simulink subsystem "ButtonStatesRF"  (R2023b) (continued)
== stateflow chart ButtonStatesRF/ButtonStates/buttonFlowChart == (continued)
                  Flow.ButtonTriggeredMenu.Menu.MainSubMenu.SettingsSubMenu.FavoritesSubMenu.Slot3Menu.Slot3FavoritesMenu.Slot3Connectivity -> Flow.ButtonTriggeredMenu.Menu.MainSubMenu.SettingsSubMenu.FavoritesSubMenu.Slot3Menu.Slot3FavoritesMenu.Slot3Odometer [order 1] : UP
                  (junction 6615) -> Flow.ButtonTriggeredMenu.Menu.MainSubMenu.SettingsSubMenu.FavoritesSubMenu.Slot3Menu.Slot3FavoritesMenu.Slot3Connectivity
                  Flow.ButtonTriggeredMenu.Menu.MainSubMenu.SettingsSubMenu.FavoritesSubMenu.Slot3Menu.Slot3FavoritesMenu.Slot3Connectivity -> (junction 6614) [order 2] : DOWN
                state favSlot3Updater(currState) [function]
                  (default) -> (junction 6665) : %{tempSlotSel  = eStateName.FAVORITES_TRIP_DISTANCE_1;}
                  (junction 6665) -> (junction 6666) [order 1] : [currState == favSlot1Sel ]
                  (junction 6666) -> (junction 6667) : { favSlot1Sel = favSlot3Sel;
                      | BLU_FnSaveSlot1Fav(favSlot1Sel);}
                  (junction 6665) -> (junction 6668) [order 2]
                  (junction 6667) -> (junction 6670)
                  (junction 6668) -> (junction 6669) [order 1] : [currState == favSlot2Sel]
                  (junction 6669) -> (junction 6670) : {favSlot2Sel = favSlot3Sel;
                      | BLU_FnSaveSlot2Fav(favSlot2Sel);}
                  (junction 6668) -> (junction 6671) [order 2]
                  (junction 6670) -> (junction 6673)
                  (junction 6671) -> (junction 6672) [order 1] : [currState == favSlot4Sel ]
                  (junction 6672) -> (junction 6673) : {favSlot4Sel = favSlot3Sel;
                      | BLU_FnSaveSlot4Fav(favSlot4Sel);}
                  (junction 6671) -> (junction 6674) [order 2]
                  (junction 6673) -> (junction 6675)
                  (junction 6675) -> (junction 6674)
                  (junction 6674) -> (junction 6676) : {favSlot3Sel = currState;
                      | BLU_FnSaveSlot3Fav(favSlot3Sel);}
                state Slot3FavoritesMenu1 [commented out]
                  state Slot3TripDistance [commented out]
                    en:
                    currentState = eStateName.FAVORITES_TRIP_DISTANCE_1;
                  state Slot3AverageSpeed [commented out]
                    en:
                    currentState = eStateName.FAVORITES_AVERAGE_SPEED_1;
                  state Slot3AverageFuelCons [commented out]
                    en:
                    currentState = eStateName.FAVORITES_AVERAGE_FUEL_CONS_1;
                  state Slot3TripTime [commented out]
                    en:
                    currentState = eStateName.FAVORITES_TRIP_TIME_1;
                  state Slot3AverageTripDistance [commented out]
                    en:
                    currentState = eStateName.FAVORITES_TRIP_DISTANCE_2;
                  state Slot3AverageSpeed1 [commented out]
                    en:
                    currentState = eStateName.FAVORITES_AVERAGE_SPEED_2;
                  state Slot3AverageFuelCons1 [commented out]
                    en:
                    currentState = eStateName.FAVORITES_AVERAGE_FUEL_CONS_2;
                  state Slot3TripTime2 [commented out]
                    en:
                    currentState = eStateName.FAVORITES_TRIP_TIME_2;
                  state Slot3FuelRange [commented out]
                    en:
                    currentState = eStateName.FAVORITES_FUEL_RANGE;
                  state Slot3Date [commented out]
                    en:
                    currentState = eStateName.FAVORITES_DATE;
                  state Slot3Battery [commented out]
                    en:
                    currentState = eStateName.FAVORITES_BATTERY;
                  state Slot3WaterTemperature [commented out]
                    en:
                    currentState = eStateName.FAVORITES_WATER_TEMPERATURE;
                  state Slot3Odometer [commented out]
                    en:
                    currentState = eStateName.FAVORITES_ODOMETER;
                  state Slot3Connectivity [commented out]
                    en:
                    currentState = eStateName.FAVORITES_CONNECTIVITY;
                  (default) -> (junction 8899) [commented out]
                  (junction 8899) -> (junction 8897) [commented out] : [favSlot3Sel ==eStateName.FAVORITES_TRIP_DISTANCE_1 ]
                  (junction 8897) -> Flow.ButtonTriggeredMenu.Menu.MainSubMenu.SettingsSubMenu.FavoritesSubMenu.Slot3Menu.Slot3FavoritesMenu1.Slot3TripDistance [commented out]
                  Flow.ButtonTriggeredMenu.Menu.MainSubMenu.SettingsSubMenu.FavoritesSubMenu.Slot3Menu.Slot3FavoritesMenu1.Slot3TripDistance -> (junction 8898) [commented out] : UP
                  (junction 8898) -> (junction 8913) [commented out]
                  (junction 8899) -> (junction 8900) [commented out]
                  (junction 8914) -> (junction 8897) [commented out]
                  Flow.ButtonTriggeredMenu.Menu.MainSubMenu.SettingsSubMenu.FavoritesSubMenu.Slot3Menu.Slot3FavoritesMenu1.Slot3TripDistance -> Flow.ButtonTriggeredMenu.Menu.MainSubMenu.SettingsSubMenu.FavoritesSubMenu.Slot3Menu.Slot3FavoritesMenu1.Slot3AverageSpeed [commented out] : DOWN
                  Flow.ButtonTriggeredMenu.Menu.MainSubMenu.SettingsSubMenu.FavoritesSubMenu.Slot3Menu.Slot3FavoritesMenu1.Slot3AverageSpeed -> Flow.ButtonTriggeredMenu.Menu.MainSubMenu.SettingsSubMenu.FavoritesSubMenu.Slot3Menu.Slot3FavoritesMenu1.Slot3TripDistance [commented out] : UP
                  (junction 8900) -> Flow.ButtonTriggeredMenu.Menu.MainSubMenu.SettingsSubMenu.FavoritesSubMenu.Slot3Menu.Slot3FavoritesMenu1.Slot3AverageSpeed [commented out] : [favSlot3Sel ==eStateName.FAVORITES_AVERAGE_SPEED_1 ]
                  (junction 8900) -> (junction 8901) [commented out]
                  Flow.ButtonTriggeredMenu.Menu.MainSubMenu.SettingsSubMenu.FavoritesSubMenu.Slot3Menu.Slot3FavoritesMenu1.Slot3AverageSpeed -> Flow.ButtonTriggeredMenu.Menu.MainSubMenu.SettingsSubMenu.FavoritesSubMenu.Slot3Menu.Slot3FavoritesMenu1.Slot3AverageFuelCons [commented out] : DOWN
                  Flow.ButtonTriggeredMenu.Menu.MainSubMenu.SettingsSubMenu.FavoritesSubMenu.Slot3Menu.Slot3FavoritesMenu1.Slot3AverageFuelCons -> Flow.ButtonTriggeredMenu.Menu.MainSubMenu.SettingsSubMenu.FavoritesSubMenu.Slot3Menu.Slot3FavoritesMenu1.Slot3AverageSpeed [commented out] : UP
                  (junction 8901) -> Flow.ButtonTriggeredMenu.Menu.MainSubMenu.SettingsSubMenu.FavoritesSubMenu.Slot3Menu.Slot3FavoritesMenu1.Slot3AverageFuelCons [commented out] : [favSlot3Sel ==eStateName.FAVORITES_AVERAGE_FUEL_CONS_1 ]
                  (junction 8901) -> (junction 8902) [commented out]
                  Flow.ButtonTriggeredMenu.Menu.MainSubMenu.SettingsSubMenu.FavoritesSubMenu.Slot3Menu.Slot3FavoritesMenu1.Slot3AverageFuelCons -> Flow.ButtonTriggeredMenu.Menu.MainSubMenu.SettingsSubMenu.FavoritesSubMenu.Slot3Menu.Slot3FavoritesMenu1.Slot3TripTime [commented out] : DOWN
                  Flow.ButtonTriggeredMenu.Menu.MainSubMenu.SettingsSubMenu.FavoritesSubMenu.Slot3Menu.Slot3FavoritesMenu1.Slot3TripTime -> Flow.ButtonTriggeredMenu.Menu.MainSubMenu.SettingsSubMenu.FavoritesSubMenu.Slot3Menu.Slot3FavoritesMenu1.Slot3AverageFuelCons [commented out] : UP
                  (junction 8902) -> Flow.ButtonTriggeredMenu.Menu.MainSubMenu.SettingsSubMenu.FavoritesSubMenu.Slot3Menu.Slot3FavoritesMenu1.Slot3TripTime [commented out] : [favSlot3Sel ==eStateName.FAVORITES_TRIP_TIME_1 ]
                  (junction 8902) -> (junction 8903) [commented out]
                  Flow.ButtonTriggeredMenu.Menu.MainSubMenu.SettingsSubMenu.FavoritesSubMenu.Slot3Menu.Slot3FavoritesMenu1.Slot3TripTime -> Flow.ButtonTriggeredMenu.Menu.MainSubMenu.SettingsSubMenu.FavoritesSubMenu.Slot3Menu.Slot3FavoritesMenu1.Slot3AverageTripDistance [commented out] : DOWN
                  Flow.ButtonTriggeredMenu.Menu.MainSubMenu.SettingsSubMenu.FavoritesSubMenu.Slot3Menu.Slot3FavoritesMenu1.Slot3AverageTripDistance -> Flow.ButtonTriggeredMenu.Menu.MainSubMenu.SettingsSubMenu.FavoritesSubMenu.Slot3Menu.Slot3FavoritesMenu1.Slot3TripTime [commented out] : UP
                  (junction 8903) -> Flow.ButtonTriggeredMenu.Menu.MainSubMenu.SettingsSubMenu.FavoritesSubMenu.Slot3Menu.Slot3FavoritesMenu1.Slot3AverageTripDistance [commented out] : [favSlot3Sel ==eStateName.FAVORITES_TRIP_DISTANCE_2 ]
                  (junction 8903) -> (junction 8904) [commented out]
                  Flow.ButtonTriggeredMenu.Menu.MainSubMenu.SettingsSubMenu.FavoritesSubMenu.Slot3Menu.Slot3FavoritesMenu1.Slot3AverageTripDistance -> Flow.ButtonTriggeredMenu.Menu.MainSubMenu.SettingsSubMenu.FavoritesSubMenu.Slot3Menu.Slot3FavoritesMenu1.Slot3AverageSpeed1 [commented out] : DOWN
                  Flow.ButtonTriggeredMenu.Menu.MainSubMenu.SettingsSubMenu.FavoritesSubMenu.Slot3Menu.Slot3FavoritesMenu1.Slot3AverageSpeed1 -> Flow.ButtonTriggeredMenu.Menu.MainSubMenu.SettingsSubMenu.FavoritesSubMenu.Slot3Menu.Slot3FavoritesMenu1.Slot3AverageTripDistance [commented out] : UP
                  (junction 8904) -> Flow.ButtonTriggeredMenu.Menu.MainSubMenu.SettingsSubMenu.FavoritesSubMenu.Slot3Menu.Slot3FavoritesMenu1.Slot3AverageSpeed1 [commented out] : [favSlot3Sel ==eStateName.FAVORITES_AVERAGE_SPEED_2 ]
                  (junction 8904) -> (junction 8905) [commented out]
                  Flow.ButtonTriggeredMenu.Menu.MainSubMenu.SettingsSubMenu.FavoritesSubMenu.Slot3Menu.Slot3FavoritesMenu1.Slot3AverageSpeed1 -> Flow.ButtonTriggeredMenu.Menu.MainSubMenu.SettingsSubMenu.FavoritesSubMenu.Slot3Menu.Slot3FavoritesMenu1.Slot3AverageFuelCons1 [commented out] : DOWN
                  Flow.ButtonTriggeredMenu.Menu.MainSubMenu.SettingsSubMenu.FavoritesSubMenu.Slot3Menu.Slot3FavoritesMenu1.Slot3AverageFuelCons1 -> Flow.ButtonTriggeredMenu.Menu.MainSubMenu.SettingsSubMenu.FavoritesSubMenu.Slot3Menu.Slot3FavoritesMenu1.Slot3AverageSpeed1 [commented out] : UP
                  (junction 8905) -> Flow.ButtonTriggeredMenu.Menu.MainSubMenu.SettingsSubMenu.FavoritesSubMenu.Slot3Menu.Slot3FavoritesMenu1.Slot3AverageFuelCons1 [commented out] : [favSlot3Sel ==eStateName.FAVORITES_AVERAGE_FUEL_CONS_2 ]
                  (junction 8905) -> (junction 8906) [commented out]
                  Flow.ButtonTriggeredMenu.Menu.MainSubMenu.SettingsSubMenu.FavoritesSubMenu.Slot3Menu.Slot3FavoritesMenu1.Slot3AverageFuelCons1 -> Flow.ButtonTriggeredMenu.Menu.MainSubMenu.SettingsSubMenu.FavoritesSubMenu.Slot3Menu.Slot3FavoritesMenu1.Slot3TripTime2 [commented out] : DOWN
                  Flow.ButtonTriggeredMenu.Menu.MainSubMenu.SettingsSubMenu.FavoritesSubMenu.Slot3Menu.Slot3FavoritesMenu1.Slot3TripTime2 -> Flow.ButtonTriggeredMenu.Menu.MainSubMenu.SettingsSubMenu.FavoritesSubMenu.Slot3Menu.Slot3FavoritesMenu1.Slot3AverageFuelCons1 [commented out] : UP
                  (junction 8906) -> Flow.ButtonTriggeredMenu.Menu.MainSubMenu.SettingsSubMenu.FavoritesSubMenu.Slot3Menu.Slot3FavoritesMenu1.Slot3TripTime2 [commented out] : [favSlot3Sel ==eStateName.FAVORITES_TRIP_TIME_2 ]
                  (junction 8906) -> (junction 8907) [commented out]
                  Flow.ButtonTriggeredMenu.Menu.MainSubMenu.SettingsSubMenu.FavoritesSubMenu.Slot3Menu.Slot3FavoritesMenu1.Slot3TripTime2 -> Flow.ButtonTriggeredMenu.Menu.MainSubMenu.SettingsSubMenu.FavoritesSubMenu.Slot3Menu.Slot3FavoritesMenu1.Slot3FuelRange [commented out] : DOWN
                  Flow.ButtonTriggeredMenu.Menu.MainSubMenu.SettingsSubMenu.FavoritesSubMenu.Slot3Menu.Slot3FavoritesMenu1.Slot3FuelRange -> Flow.ButtonTriggeredMenu.Menu.MainSubMenu.SettingsSubMenu.FavoritesSubMenu.Slot3Menu.Slot3FavoritesMenu1.Slot3TripTime2 [commented out] : UP
                  (junction 8907) -> Flow.ButtonTriggeredMenu.Menu.MainSubMenu.SettingsSubMenu.FavoritesSubMenu.Slot3Menu.Slot3FavoritesMenu1.Slot3FuelRange [commented out] : [favSlot3Sel ==eStateName.FAVORITES_FUEL_RANGE ]
                  (junction 8907) -> (junction 8908) [commented out]
                  Flow.ButtonTriggeredMenu.Menu.MainSubMenu.SettingsSubMenu.FavoritesSubMenu.Slot3Menu.Slot3FavoritesMenu1.Slot3FuelRange -> Flow.ButtonTriggeredMenu.Menu.MainSubMenu.SettingsSubMenu.FavoritesSubMenu.Slot3Menu.Slot3FavoritesMenu1.Slot3Date [commented out] : DOWN
                  Flow.ButtonTriggeredMenu.Menu.MainSubMenu.SettingsSubMenu.FavoritesSubMenu.Slot3Menu.Slot3FavoritesMenu1.Slot3Date -> Flow.ButtonTriggeredMenu.Menu.MainSubMenu.SettingsSubMenu.FavoritesSubMenu.Slot3Menu.Slot3FavoritesMenu1.Slot3FuelRange [commented out] : UP
                  (junction 8908) -> Flow.ButtonTriggeredMenu.Menu.MainSubMenu.SettingsSubMenu.FavoritesSubMenu.Slot3Menu.Slot3FavoritesMenu1.Slot3Date [commented out] : [favSlot3Sel ==eStateName.FAVORITES_DATE ]
                  (junction 8908) -> (junction 8909) [commented out]
                  Flow.ButtonTriggeredMenu.Menu.MainSubMenu.SettingsSubMenu.FavoritesSubMenu.Slot3Menu.Slot3FavoritesMenu1.Slot3Date -> Flow.ButtonTriggeredMenu.Menu.MainSubMenu.SettingsSubMenu.FavoritesSubMenu.Slot3Menu.Slot3FavoritesMenu1.Slot3Battery [commented out] : DOWN
                  Flow.ButtonTriggeredMenu.Menu.MainSubMenu.SettingsSubMenu.FavoritesSubMenu.Slot3Menu.Slot3FavoritesMenu1.Slot3Battery -> Flow.ButtonTriggeredMenu.Menu.MainSubMenu.SettingsSubMenu.FavoritesSubMenu.Slot3Menu.Slot3FavoritesMenu1.Slot3Date [commented out] : UP
                  (junction 8909) -> Flow.ButtonTriggeredMenu.Menu.MainSubMenu.SettingsSubMenu.FavoritesSubMenu.Slot3Menu.Slot3FavoritesMenu1.Slot3Battery [commented out] : [favSlot3Sel ==eStateName.FAVORITES_BATTERY ]
                  (junction 8909) -> (junction 8910) [commented out]
                  Flow.ButtonTriggeredMenu.Menu.MainSubMenu.SettingsSubMenu.FavoritesSubMenu.Slot3Menu.Slot3FavoritesMenu1.Slot3Battery -> Flow.ButtonTriggeredMenu.Menu.MainSubMenu.SettingsSubMenu.FavoritesSubMenu.Slot3Menu.Slot3FavoritesMenu1.Slot3WaterTemperature [commented out] : DOWN
                  Flow.ButtonTriggeredMenu.Menu.MainSubMenu.SettingsSubMenu.FavoritesSubMenu.Slot3Menu.Slot3FavoritesMenu1.Slot3WaterTemperature -> Flow.ButtonTriggeredMenu.Menu.MainSubMenu.SettingsSubMenu.FavoritesSubMenu.Slot3Menu.Slot3FavoritesMenu1.Slot3Battery [commented out] : UP
                  (junction 8910) -> Flow.ButtonTriggeredMenu.Menu.MainSubMenu.SettingsSubMenu.FavoritesSubMenu.Slot3Menu.Slot3FavoritesMenu1.Slot3WaterTemperature [commented out] : [favSlot3Sel ==eStateName.FAVORITES_WATER_TEMPERATURE ]
                  (junction 8910) -> (junction 8911) [commented out]
                  Flow.ButtonTriggeredMenu.Menu.MainSubMenu.SettingsSubMenu.FavoritesSubMenu.Slot3Menu.Slot3FavoritesMenu1.Slot3WaterTemperature -> Flow.ButtonTriggeredMenu.Menu.MainSubMenu.SettingsSubMenu.FavoritesSubMenu.Slot3Menu.Slot3FavoritesMenu1.Slot3Odometer [commented out] : DOWN
                  Flow.ButtonTriggeredMenu.Menu.MainSubMenu.SettingsSubMenu.FavoritesSubMenu.Slot3Menu.Slot3FavoritesMenu1.Slot3Odometer -> Flow.ButtonTriggeredMenu.Menu.MainSubMenu.SettingsSubMenu.FavoritesSubMenu.Slot3Menu.Slot3FavoritesMenu1.Slot3WaterTemperature [commented out] : UP
                  (junction 8911) -> Flow.ButtonTriggeredMenu.Menu.MainSubMenu.SettingsSubMenu.FavoritesSubMenu.Slot3Menu.Slot3FavoritesMenu1.Slot3Odometer [commented out] : [favSlot3Sel ==eStateName.FAVORITES_ODOMETER ]
                  (junction 8911) -> (junction 8912) [commented out]
                  Flow.ButtonTriggeredMenu.Menu.MainSubMenu.SettingsSubMenu.FavoritesSubMenu.Slot3Menu.Slot3FavoritesMenu1.Slot3Odometer -> Flow.ButtonTriggeredMenu.Menu.MainSubMenu.SettingsSubMenu.FavoritesSubMenu.Slot3Menu.Slot3FavoritesMenu1.Slot3Connectivity [commented out] : DOWN
                  Flow.ButtonTriggeredMenu.Menu.MainSubMenu.SettingsSubMenu.FavoritesSubMenu.Slot3Menu.Slot3FavoritesMenu1.Slot3Connectivity -> Flow.ButtonTriggeredMenu.Menu.MainSubMenu.SettingsSubMenu.FavoritesSubMenu.Slot3Menu.Slot3FavoritesMenu1.Slot3Odometer [commented out] : UP
                  (junction 8912) -> Flow.ButtonTriggeredMenu.Menu.MainSubMenu.SettingsSubMenu.FavoritesSubMenu.Slot3Menu.Slot3FavoritesMenu1.Slot3Connectivity [commented out]
                  (junction 8913) -> Flow.ButtonTriggeredMenu.Menu.MainSubMenu.SettingsSubMenu.FavoritesSubMenu.Slot3Menu.Slot3FavoritesMenu1.Slot3Connectivity [commented out]
                  Flow.ButtonTriggeredMenu.Menu.MainSubMenu.SettingsSubMenu.FavoritesSubMenu.Slot3Menu.Slot3FavoritesMenu1.Slot3Connectivity -> (junction 8914) [commented out] : DOWN
                6604 -> Slot3FavoritesMenu
                Slot3FavoritesMenu -> 6605 [order 2] : EXIT
                (junction 6603) -> 6605 : {favSlot3Updater(currentState);}
                Slot3FavoritesMenu -> (junction 6603) [order 1] : SET
              state Slot4Menu
                state Slot4FavoritesMenu
                  (history)
                  state Slot4TripDistance
                    en:
                    currentState = eStateName.FAVORITES_TRIP_DISTANCE_1;
                  state Slot4AverageSpeed
                    en:
                    currentState = eStateName.FAVORITES_AVERAGE_SPEED_1;
                  state Slot4AverageFuelCons
                    en:
                    currentState = eStateName.FAVORITES_AVERAGE_FUEL_CONS_1;
                  state Slot4TripTime
                    en:
                    currentState = eStateName.FAVORITES_TRIP_TIME_1;
                  state Slot4AverageTripDistance
                    en:
                    currentState = eStateName.FAVORITES_TRIP_DISTANCE_2;
                  state Slot4AverageSpeed1
                    en:
                    currentState = eStateName.FAVORITES_AVERAGE_SPEED_2;
                  state Slot4AverageFuelCons1
                    en:
                    currentState = eStateName.FAVORITES_AVERAGE_FUEL_CONS_2;
                  state Slot4TripTime1
                    en:
                    currentState = eStateName.FAVORITES_TRIP_TIME_2;
                  state Slot4FuelRange
                    en:
                    currentState = eStateName.FAVORITES_FUEL_RANGE;
                  state Slot4Date
                    en:
                    currentState = eStateName.FAVORITES_DATE;
                  state Slot4Battery
                    en:
                    currentState = eStateName.FAVORITES_BATTERY;
                  state Slot4WaterTemperature
                    en:
                    currentState = eStateName.FAVORITES_WATER_TEMPERATURE;
                  state Slot4Odometer
                    en:
                    currentState = eStateName.FAVORITES_ODOMETER;
                  state Slot1Connectivity
                    en:
                    currentState = eStateName.FAVORITES_CONNECTIVITY;
                  (default) -> (junction 6702)
                  (junction 6702) -> Flow.ButtonTriggeredMenu.Menu.MainSubMenu.SettingsSubMenu.FavoritesSubMenu.Slot4Menu.Slot4FavoritesMenu.Slot4TripDistance
                  Flow.ButtonTriggeredMenu.Menu.MainSubMenu.SettingsSubMenu.FavoritesSubMenu.Slot4Menu.Slot4FavoritesMenu.Slot4TripDistance -> (junction 6703) [order 2] : UP
                  (junction 6704) -> (junction 6702)
                  (junction 6703) -> (junction 6705)
                  Flow.ButtonTriggeredMenu.Menu.MainSubMenu.SettingsSubMenu.FavoritesSubMenu.Slot4Menu.Slot4FavoritesMenu.Slot4TripDistance -> Flow.ButtonTriggeredMenu.Menu.MainSubMenu.SettingsSubMenu.FavoritesSubMenu.Slot4Menu.Slot4FavoritesMenu.Slot4AverageSpeed [order 1] : DOWN
                  Flow.ButtonTriggeredMenu.Menu.MainSubMenu.SettingsSubMenu.FavoritesSubMenu.Slot4Menu.Slot4FavoritesMenu.Slot4AverageSpeed -> Flow.ButtonTriggeredMenu.Menu.MainSubMenu.SettingsSubMenu.FavoritesSubMenu.Slot4Menu.Slot4FavoritesMenu.Slot4TripDistance [order 1] : UP
                  Flow.ButtonTriggeredMenu.Menu.MainSubMenu.SettingsSubMenu.FavoritesSubMenu.Slot4Menu.Slot4FavoritesMenu.Slot4AverageSpeed -> Flow.ButtonTriggeredMenu.Menu.MainSubMenu.SettingsSubMenu.FavoritesSubMenu.Slot4Menu.Slot4FavoritesMenu.Slot4AverageFuelCons [order 2] : DOWN
                  Flow.ButtonTriggeredMenu.Menu.MainSubMenu.SettingsSubMenu.FavoritesSubMenu.Slot4Menu.Slot4FavoritesMenu.Slot4AverageFuelCons -> Flow.ButtonTriggeredMenu.Menu.MainSubMenu.SettingsSubMenu.FavoritesSubMenu.Slot4Menu.Slot4FavoritesMenu.Slot4AverageSpeed [order 1] : UP
                  Flow.ButtonTriggeredMenu.Menu.MainSubMenu.SettingsSubMenu.FavoritesSubMenu.Slot4Menu.Slot4FavoritesMenu.Slot4AverageFuelCons -> Flow.ButtonTriggeredMenu.Menu.MainSubMenu.SettingsSubMenu.FavoritesSubMenu.Slot4Menu.Slot4FavoritesMenu.Slot4TripTime [order 2] : DOWN
                  Flow.ButtonTriggeredMenu.Menu.MainSubMenu.SettingsSubMenu.FavoritesSubMenu.Slot4Menu.Slot4FavoritesMenu.Slot4TripTime -> Flow.ButtonTriggeredMenu.Menu.MainSubMenu.SettingsSubMenu.FavoritesSubMenu.Slot4Menu.Slot4FavoritesMenu.Slot4AverageFuelCons [order 1] : UP
                  Flow.ButtonTriggeredMenu.Menu.MainSubMenu.SettingsSubMenu.FavoritesSubMenu.Slot4Menu.Slot4FavoritesMenu.Slot4TripTime -> Flow.ButtonTriggeredMenu.Menu.MainSubMenu.SettingsSubMenu.FavoritesSubMenu.Slot4Menu.Slot4FavoritesMenu.Slot4AverageTripDistance [order 2] : DOWN
                  Flow.ButtonTriggeredMenu.Menu.MainSubMenu.SettingsSubMenu.FavoritesSubMenu.Slot4Menu.Slot4FavoritesMenu.Slot4AverageTripDistance -> Flow.ButtonTriggeredMenu.Menu.MainSubMenu.SettingsSubMenu.FavoritesSubMenu.Slot4Menu.Slot4FavoritesMenu.Slot4TripTime [order 1] : UP
                  Flow.ButtonTriggeredMenu.Menu.MainSubMenu.SettingsSubMenu.FavoritesSubMenu.Slot4Menu.Slot4FavoritesMenu.Slot4AverageTripDistance -> Flow.ButtonTriggeredMenu.Menu.MainSubMenu.SettingsSubMenu.FavoritesSubMenu.Slot4Menu.Slot4FavoritesMenu.Slot4AverageSpeed1 [order 2] : DOWN
                  Flow.ButtonTriggeredMenu.Menu.MainSubMenu.SettingsSubMenu.FavoritesSubMenu.Slot4Menu.Slot4FavoritesMenu.Slot4AverageSpeed1 -> Flow.ButtonTriggeredMenu.Menu.MainSubMenu.SettingsSubMenu.FavoritesSubMenu.Slot4Menu.Slot4FavoritesMenu.Slot4AverageTripDistance [order 1] : UP
                  Flow.ButtonTriggeredMenu.Menu.MainSubMenu.SettingsSubMenu.FavoritesSubMenu.Slot4Menu.Slot4FavoritesMenu.Slot4AverageSpeed1 -> Flow.ButtonTriggeredMenu.Menu.MainSubMenu.SettingsSubMenu.FavoritesSubMenu.Slot4Menu.Slot4FavoritesMenu.Slot4AverageFuelCons1 [order 2] : DOWN
                  Flow.ButtonTriggeredMenu.Menu.MainSubMenu.SettingsSubMenu.FavoritesSubMenu.Slot4Menu.Slot4FavoritesMenu.Slot4AverageFuelCons1 -> Flow.ButtonTriggeredMenu.Menu.MainSubMenu.SettingsSubMenu.FavoritesSubMenu.Slot4Menu.Slot4FavoritesMenu.Slot4AverageSpeed1 [order 1] : UP
                  Flow.ButtonTriggeredMenu.Menu.MainSubMenu.SettingsSubMenu.FavoritesSubMenu.Slot4Menu.Slot4FavoritesMenu.Slot4AverageFuelCons1 -> Flow.ButtonTriggeredMenu.Menu.MainSubMenu.SettingsSubMenu.FavoritesSubMenu.Slot4Menu.Slot4FavoritesMenu.Slot4TripTime1 [order 2] : DOWN
                  Flow.ButtonTriggeredMenu.Menu.MainSubMenu.SettingsSubMenu.FavoritesSubMenu.Slot4Menu.Slot4FavoritesMenu.Slot4TripTime1 -> Flow.ButtonTriggeredMenu.Menu.MainSubMenu.SettingsSubMenu.FavoritesSubMenu.Slot4Menu.Slot4FavoritesMenu.Slot4AverageFuelCons1 [order 1] : UP
                  Flow.ButtonTriggeredMenu.Menu.MainSubMenu.SettingsSubMenu.FavoritesSubMenu.Slot4Menu.Slot4FavoritesMenu.Slot4TripTime1 -> Flow.ButtonTriggeredMenu.Menu.MainSubMenu.SettingsSubMenu.FavoritesSubMenu.Slot4Menu.Slot4FavoritesMenu.Slot4FuelRange [order 2] : DOWN
                  Flow.ButtonTriggeredMenu.Menu.MainSubMenu.SettingsSubMenu.FavoritesSubMenu.Slot4Menu.Slot4FavoritesMenu.Slot4FuelRange -> Flow.ButtonTriggeredMenu.Menu.MainSubMenu.SettingsSubMenu.FavoritesSubMenu.Slot4Menu.Slot4FavoritesMenu.Slot4TripTime1 [order 1] : UP
                  Flow.ButtonTriggeredMenu.Menu.MainSubMenu.SettingsSubMenu.FavoritesSubMenu.Slot4Menu.Slot4FavoritesMenu.Slot4FuelRange -> Flow.ButtonTriggeredMenu.Menu.MainSubMenu.SettingsSubMenu.FavoritesSubMenu.Slot4Menu.Slot4FavoritesMenu.Slot4Date [order 2] : DOWN
                  Flow.ButtonTriggeredMenu.Menu.MainSubMenu.SettingsSubMenu.FavoritesSubMenu.Slot4Menu.Slot4FavoritesMenu.Slot4Date -> Flow.ButtonTriggeredMenu.Menu.MainSubMenu.SettingsSubMenu.FavoritesSubMenu.Slot4Menu.Slot4FavoritesMenu.Slot4FuelRange [order 1] : UP
                  Flow.ButtonTriggeredMenu.Menu.MainSubMenu.SettingsSubMenu.FavoritesSubMenu.Slot4Menu.Slot4FavoritesMenu.Slot4Date -> Flow.ButtonTriggeredMenu.Menu.MainSubMenu.SettingsSubMenu.FavoritesSubMenu.Slot4Menu.Slot4FavoritesMenu.Slot4Battery [order 2] : DOWN
                  Flow.ButtonTriggeredMenu.Menu.MainSubMenu.SettingsSubMenu.FavoritesSubMenu.Slot4Menu.Slot4FavoritesMenu.Slot4Battery -> Flow.ButtonTriggeredMenu.Menu.MainSubMenu.SettingsSubMenu.FavoritesSubMenu.Slot4Menu.Slot4FavoritesMenu.Slot4Date [order 1] : UP
                  Flow.ButtonTriggeredMenu.Menu.MainSubMenu.SettingsSubMenu.FavoritesSubMenu.Slot4Menu.Slot4FavoritesMenu.Slot4Battery -> Flow.ButtonTriggeredMenu.Menu.MainSubMenu.SettingsSubMenu.FavoritesSubMenu.Slot4Menu.Slot4FavoritesMenu.Slot4WaterTemperature [order 2] : DOWN
                  Flow.ButtonTriggeredMenu.Menu.MainSubMenu.SettingsSubMenu.FavoritesSubMenu.Slot4Menu.Slot4FavoritesMenu.Slot4WaterTemperature -> Flow.ButtonTriggeredMenu.Menu.MainSubMenu.SettingsSubMenu.FavoritesSubMenu.Slot4Menu.Slot4FavoritesMenu.Slot4Battery [order 1] : UP
                  Flow.ButtonTriggeredMenu.Menu.MainSubMenu.SettingsSubMenu.FavoritesSubMenu.Slot4Menu.Slot4FavoritesMenu.Slot4WaterTemperature -> Flow.ButtonTriggeredMenu.Menu.MainSubMenu.SettingsSubMenu.FavoritesSubMenu.Slot4Menu.Slot4FavoritesMenu.Slot4Odometer [order 2] : DOWN
                  Flow.ButtonTriggeredMenu.Menu.MainSubMenu.SettingsSubMenu.FavoritesSubMenu.Slot4Menu.Slot4FavoritesMenu.Slot4Odometer -> Flow.ButtonTriggeredMenu.Menu.MainSubMenu.SettingsSubMenu.FavoritesSubMenu.Slot4Menu.Slot4FavoritesMenu.Slot4WaterTemperature [order 1] : UP
                  Flow.ButtonTriggeredMenu.Menu.MainSubMenu.SettingsSubMenu.FavoritesSubMenu.Slot4Menu.Slot4FavoritesMenu.Slot4Odometer -> Flow.ButtonTriggeredMenu.Menu.MainSubMenu.SettingsSubMenu.FavoritesSubMenu.Slot4Menu.Slot4FavoritesMenu.Slot1Connectivity [order 2] : DOWN
                  Flow.ButtonTriggeredMenu.Menu.MainSubMenu.SettingsSubMenu.FavoritesSubMenu.Slot4Menu.Slot4FavoritesMenu.Slot1Connectivity -> Flow.ButtonTriggeredMenu.Menu.MainSubMenu.SettingsSubMenu.FavoritesSubMenu.Slot4Menu.Slot4FavoritesMenu.Slot4Odometer [order 1] : UP
                  Flow.ButtonTriggeredMenu.Menu.MainSubMenu.SettingsSubMenu.FavoritesSubMenu.Slot4Menu.Slot4FavoritesMenu.Slot1Connectivity -> (junction 6704) [order 2] : DOWN
                  (junction 6705) -> Flow.ButtonTriggeredMenu.Menu.MainSubMenu.SettingsSubMenu.FavoritesSubMenu.Slot4Menu.Slot4FavoritesMenu.Slot1Connectivity
                state favSlot4Updater(currState) [function]
                  (default) -> (junction 6755) : %{tempSlotSel  = eStateName.FAVORITES_TRIP_DISTANCE_1;}
                  (junction 6755) -> (junction 6756) [order 1] : [currState == favSlot1Sel ]
                  (junction 6756) -> (junction 6757) : { favSlot1Sel = favSlot4Sel;
                      | BLU_FnSaveSlot1Fav(favSlot1Sel);}
                  (junction 6755) -> (junction 6758) [order 2]
                  (junction 6757) -> (junction 6760)
                  (junction 6758) -> (junction 6759) [order 1] : [currState == favSlot2Sel]
                  (junction 6759) -> (junction 6760) : {favSlot2Sel = favSlot4Sel;
                      | BLU_FnSaveSlot2Fav(favSlot2Sel);}
                  (junction 6758) -> (junction 6761) [order 2]
                  (junction 6760) -> (junction 6763)
                  (junction 6761) -> (junction 6762) [order 1] : [currState == favSlot3Sel ]
                  (junction 6762) -> (junction 6763) : {favSlot3Sel = favSlot4Sel;
                      | BLU_FnSaveSlot3Fav(favSlot3Sel);}
                  (junction 6761) -> (junction 6764) [order 2]
                  (junction 6763) -> (junction 6765)
                  (junction 6765) -> (junction 6764)
                  (junction 6764) -> (junction 6766) : {favSlot4Sel = currState;
                      | BLU_FnSaveSlot4Fav(favSlot4Sel);}
                state Slot4FavoritesMenu1 [commented out]
                  state Slot4TripDistance [commented out]
                    en:
                    currentState = eStateName.FAVORITES_TRIP_DISTANCE_1;
                  state Slot4AverageSpeed [commented out]
                    en:
                    currentState = eStateName.FAVORITES_AVERAGE_SPEED_1;
                  state Slot4AverageFuelCons [commented out]
                    en:
                    currentState = eStateName.FAVORITES_AVERAGE_FUEL_CONS_1;
                  state Slot4TripTime [commented out]
                    en:
                    currentState = eStateName.FAVORITES_TRIP_TIME_1;
                  state Slot4AverageTripDistance [commented out]
                    en:
                    currentState = eStateName.FAVORITES_TRIP_DISTANCE_2;
                  state Slot4AverageSpeed1 [commented out]
                    en:
                    currentState = eStateName.FAVORITES_AVERAGE_SPEED_2;
                  state Slot4AverageFuelCons1 [commented out]
                    en:
                    currentState = eStateName.FAVORITES_AVERAGE_FUEL_CONS_2;
                  state Slot4TripTime1 [commented out]
                    en:
                    currentState = eStateName.FAVORITES_TRIP_TIME_2;
                  state Slot4FuelRange [commented out]
                    en:
                    currentState = eStateName.FAVORITES_FUEL_RANGE;
                  state Slot4Date [commented out]
                    en:
                    currentState = eStateName.FAVORITES_DATE;
                  state Slot4Battery [commented out]
                    en:
                    currentState = eStateName.FAVORITES_BATTERY;
                  state Slot4WaterTemperature [commented out]
                    en:
                    currentState = eStateName.FAVORITES_WATER_TEMPERATURE;
                  state Slot4Odometer [commented out]
                    en:
                    currentState = eStateName.FAVORITES_ODOMETER;
                  state Slot1Connectivity [commented out]
                    en:
                    currentState = eStateName.FAVORITES_CONNECTIVITY;
                  (default) -> (junction 8992) [commented out]
                  (junction 8992) -> (junction 8993) [commented out] : [favSlot4Sel ==eStateName.FAVORITES_TRIP_DISTANCE_1 ]
                  (junction 8993) -> Flow.ButtonTriggeredMenu.Menu.MainSubMenu.SettingsSubMenu.FavoritesSubMenu.Slot4Menu.Slot4FavoritesMenu1.Slot4TripDistance [commented out]
                  Flow.ButtonTriggeredMenu.Menu.MainSubMenu.SettingsSubMenu.FavoritesSubMenu.Slot4Menu.Slot4FavoritesMenu1.Slot4TripDistance -> (junction 8994) [commented out] : UP
                  (junction 9008) -> (junction 8993) [commented out]
                  (junction 8994) -> (junction 9009) [commented out]
                  (junction 8992) -> (junction 8995) [commented out]
                  Flow.ButtonTriggeredMenu.Menu.MainSubMenu.SettingsSubMenu.FavoritesSubMenu.Slot4Menu.Slot4FavoritesMenu1.Slot4TripDistance -> Flow.ButtonTriggeredMenu.Menu.MainSubMenu.SettingsSubMenu.FavoritesSubMenu.Slot4Menu.Slot4FavoritesMenu1.Slot4AverageSpeed [commented out] : DOWN
                  Flow.ButtonTriggeredMenu.Menu.MainSubMenu.SettingsSubMenu.FavoritesSubMenu.Slot4Menu.Slot4FavoritesMenu1.Slot4AverageSpeed -> Flow.ButtonTriggeredMenu.Menu.MainSubMenu.SettingsSubMenu.FavoritesSubMenu.Slot4Menu.Slot4FavoritesMenu1.Slot4TripDistance [commented out] : UP
                  (junction 8995) -> Flow.ButtonTriggeredMenu.Menu.MainSubMenu.SettingsSubMenu.FavoritesSubMenu.Slot4Menu.Slot4FavoritesMenu1.Slot4AverageSpeed [commented out] : [favSlot4Sel ==eStateName.FAVORITES_AVERAGE_SPEED_1 ]
                  (junction 8995) -> (junction 8996) [commented out]
                  Flow.ButtonTriggeredMenu.Menu.MainSubMenu.SettingsSubMenu.FavoritesSubMenu.Slot4Menu.Slot4FavoritesMenu1.Slot4AverageSpeed -> Flow.ButtonTriggeredMenu.Menu.MainSubMenu.SettingsSubMenu.FavoritesSubMenu.Slot4Menu.Slot4FavoritesMenu1.Slot4AverageFuelCons [commented out] : DOWN
                  Flow.ButtonTriggeredMenu.Menu.MainSubMenu.SettingsSubMenu.FavoritesSubMenu.Slot4Menu.Slot4FavoritesMenu1.Slot4AverageFuelCons -> Flow.ButtonTriggeredMenu.Menu.MainSubMenu.SettingsSubMenu.FavoritesSubMenu.Slot4Menu.Slot4FavoritesMenu1.Slot4AverageSpeed [commented out] : UP
                  (junction 8996) -> Flow.ButtonTriggeredMenu.Menu.MainSubMenu.SettingsSubMenu.FavoritesSubMenu.Slot4Menu.Slot4FavoritesMenu1.Slot4AverageFuelCons [commented out] : [favSlot4Sel ==eStateName.FAVORITES_AVERAGE_FUEL_CONS_1 ]
                  (junction 8996) -> (junction 8997) [commented out]
                  Flow.ButtonTriggeredMenu.Menu.MainSubMenu.SettingsSubMenu.FavoritesSubMenu.Slot4Menu.Slot4FavoritesMenu1.Slot4AverageFuelCons -> Flow.ButtonTriggeredMenu.Menu.MainSubMenu.SettingsSubMenu.FavoritesSubMenu.Slot4Menu.Slot4FavoritesMenu1.Slot4TripTime [commented out] : DOWN
                  Flow.ButtonTriggeredMenu.Menu.MainSubMenu.SettingsSubMenu.FavoritesSubMenu.Slot4Menu.Slot4FavoritesMenu1.Slot4TripTime -> Flow.ButtonTriggeredMenu.Menu.MainSubMenu.SettingsSubMenu.FavoritesSubMenu.Slot4Menu.Slot4FavoritesMenu1.Slot4AverageFuelCons [commented out] : UP
                  (junction 8997) -> Flow.ButtonTriggeredMenu.Menu.MainSubMenu.SettingsSubMenu.FavoritesSubMenu.Slot4Menu.Slot4FavoritesMenu1.Slot4TripTime [commented out] : [favSlot4Sel ==eStateName.FAVORITES_TRIP_TIME_1 ]
                  (junction 8997) -> (junction 8998) [commented out]
                  Flow.ButtonTriggeredMenu.Menu.MainSubMenu.SettingsSubMenu.FavoritesSubMenu.Slot4Menu.Slot4FavoritesMenu1.Slot4TripTime -> Flow.ButtonTriggeredMenu.Menu.MainSubMenu.SettingsSubMenu.FavoritesSubMenu.Slot4Menu.Slot4FavoritesMenu1.Slot4AverageTripDistance [commented out] : DOWN
                  Flow.ButtonTriggeredMenu.Menu.MainSubMenu.SettingsSubMenu.FavoritesSubMenu.Slot4Menu.Slot4FavoritesMenu1.Slot4AverageTripDistance -> Flow.ButtonTriggeredMenu.Menu.MainSubMenu.SettingsSubMenu.FavoritesSubMenu.Slot4Menu.Slot4FavoritesMenu1.Slot4TripTime [commented out] : UP
                  (junction 8998) -> Flow.ButtonTriggeredMenu.Menu.MainSubMenu.SettingsSubMenu.FavoritesSubMenu.Slot4Menu.Slot4FavoritesMenu1.Slot4AverageTripDistance [commented out] : [favSlot4Sel ==eStateName.FAVORITES_TRIP_DISTANCE_2 ]
                  (junction 8998) -> (junction 8999) [commented out]
                  Flow.ButtonTriggeredMenu.Menu.MainSubMenu.SettingsSubMenu.FavoritesSubMenu.Slot4Menu.Slot4FavoritesMenu1.Slot4AverageTripDistance -> Flow.ButtonTriggeredMenu.Menu.MainSubMenu.SettingsSubMenu.FavoritesSubMenu.Slot4Menu.Slot4FavoritesMenu1.Slot4AverageSpeed1 [commented out] : DOWN
                  Flow.ButtonTriggeredMenu.Menu.MainSubMenu.SettingsSubMenu.FavoritesSubMenu.Slot4Menu.Slot4FavoritesMenu1.Slot4AverageSpeed1 -> Flow.ButtonTriggeredMenu.Menu.MainSubMenu.SettingsSubMenu.FavoritesSubMenu.Slot4Menu.Slot4FavoritesMenu1.Slot4AverageTripDistance [commented out] : UP
                  (junction 8999) -> Flow.ButtonTriggeredMenu.Menu.MainSubMenu.SettingsSubMenu.FavoritesSubMenu.Slot4Menu.Slot4FavoritesMenu1.Slot4AverageSpeed1 [commented out] : [favSlot4Sel ==eStateName.FAVORITES_AVERAGE_SPEED_2 ]
                  (junction 8999) -> (junction 9000) [commented out]
                  Flow.ButtonTriggeredMenu.Menu.MainSubMenu.SettingsSubMenu.FavoritesSubMenu.Slot4Menu.Slot4FavoritesMenu1.Slot4AverageSpeed1 -> Flow.ButtonTriggeredMenu.Menu.MainSubMenu.SettingsSubMenu.FavoritesSubMenu.Slot4Menu.Slot4FavoritesMenu1.Slot4AverageFuelCons1 [commented out] : DOWN
                  Flow.ButtonTriggeredMenu.Menu.MainSubMenu.SettingsSubMenu.FavoritesSubMenu.Slot4Menu.Slot4FavoritesMenu1.Slot4AverageFuelCons1 -> Flow.ButtonTriggeredMenu.Menu.MainSubMenu.SettingsSubMenu.FavoritesSubMenu.Slot4Menu.Slot4FavoritesMenu1.Slot4AverageSpeed1 [commented out] : UP
                  (junction 9000) -> Flow.ButtonTriggeredMenu.Menu.MainSubMenu.SettingsSubMenu.FavoritesSubMenu.Slot4Menu.Slot4FavoritesMenu1.Slot4AverageFuelCons1 [commented out] : [favSlot4Sel ==eStateName.FAVORITES_AVERAGE_FUEL_CONS_2 ]
                  (junction 9000) -> (junction 9001) [commented out]
                  Flow.ButtonTriggeredMenu.Menu.MainSubMenu.SettingsSubMenu.FavoritesSubMenu.Slot4Menu.Slot4FavoritesMenu1.Slot4AverageFuelCons1 -> Flow.ButtonTriggeredMenu.Menu.MainSubMenu.SettingsSubMenu.FavoritesSubMenu.Slot4Menu.Slot4FavoritesMenu1.Slot4TripTime1 [commented out] : DOWN
                  Flow.ButtonTriggeredMenu.Menu.MainSubMenu.SettingsSubMenu.FavoritesSubMenu.Slot4Menu.Slot4FavoritesMenu1.Slot4TripTime1 -> Flow.ButtonTriggeredMenu.Menu.MainSubMenu.SettingsSubMenu.FavoritesSubMenu.Slot4Menu.Slot4FavoritesMenu1.Slot4AverageFuelCons1 [commented out] : UP
                  (junction 9001) -> Flow.ButtonTriggeredMenu.Menu.MainSubMenu.SettingsSubMenu.FavoritesSubMenu.Slot4Menu.Slot4FavoritesMenu1.Slot4TripTime1 [commented out] : [favSlot4Sel ==eStateName.FAVORITES_TRIP_TIME_2 ]
                  (junction 9001) -> (junction 9002) [commented out]
                  Flow.ButtonTriggeredMenu.Menu.MainSubMenu.SettingsSubMenu.FavoritesSubMenu.Slot4Menu.Slot4FavoritesMenu1.Slot4TripTime1 -> Flow.ButtonTriggeredMenu.Menu.MainSubMenu.SettingsSubMenu.FavoritesSubMenu.Slot4Menu.Slot4FavoritesMenu1.Slot4FuelRange [commented out] : DOWN
                  Flow.ButtonTriggeredMenu.Menu.MainSubMenu.SettingsSubMenu.FavoritesSubMenu.Slot4Menu.Slot4FavoritesMenu1.Slot4FuelRange -> Flow.ButtonTriggeredMenu.Menu.MainSubMenu.SettingsSubMenu.FavoritesSubMenu.Slot4Menu.Slot4FavoritesMenu1.Slot4TripTime1 [commented out] : UP
                  (junction 9002) -> Flow.ButtonTriggeredMenu.Menu.MainSubMenu.SettingsSubMenu.FavoritesSubMenu.Slot4Menu.Slot4FavoritesMenu1.Slot4FuelRange [commented out] : [favSlot4Sel ==eStateName.FAVORITES_FUEL_RANGE ]
                  (junction 9002) -> (junction 9003) [commented out]
                  Flow.ButtonTriggeredMenu.Menu.MainSubMenu.SettingsSubMenu.FavoritesSubMenu.Slot4Menu.Slot4FavoritesMenu1.Slot4FuelRange -> Flow.ButtonTriggeredMenu.Menu.MainSubMenu.SettingsSubMenu.FavoritesSubMenu.Slot4Menu.Slot4FavoritesMenu1.Slot4Date [commented out] : DOWN
                  Flow.ButtonTriggeredMenu.Menu.MainSubMenu.SettingsSubMenu.FavoritesSubMenu.Slot4Menu.Slot4FavoritesMenu1.Slot4Date -> Flow.ButtonTriggeredMenu.Menu.MainSubMenu.SettingsSubMenu.FavoritesSubMenu.Slot4Menu.Slot4FavoritesMenu1.Slot4FuelRange [commented out] : UP
                  (junction 9003) -> Flow.ButtonTriggeredMenu.Menu.MainSubMenu.SettingsSubMenu.FavoritesSubMenu.Slot4Menu.Slot4FavoritesMenu1.Slot4Date [commented out] : [favSlot4Sel ==eStateName.FAVORITES_DATE ]
                  (junction 9003) -> (junction 9004) [commented out]
                  Flow.ButtonTriggeredMenu.Menu.MainSubMenu.SettingsSubMenu.FavoritesSubMenu.Slot4Menu.Slot4FavoritesMenu1.Slot4Date -> Flow.ButtonTriggeredMenu.Menu.MainSubMenu.SettingsSubMenu.FavoritesSubMenu.Slot4Menu.Slot4FavoritesMenu1.Slot4Battery [commented out] : DOWN
                  Flow.ButtonTriggeredMenu.Menu.MainSubMenu.SettingsSubMenu.FavoritesSubMenu.Slot4Menu.Slot4FavoritesMenu1.Slot4Battery -> Flow.ButtonTriggeredMenu.Menu.MainSubMenu.SettingsSubMenu.FavoritesSubMenu.Slot4Menu.Slot4FavoritesMenu1.Slot4Date [commented out] : UP
                  (junction 9004) -> Flow.ButtonTriggeredMenu.Menu.MainSubMenu.SettingsSubMenu.FavoritesSubMenu.Slot4Menu.Slot4FavoritesMenu1.Slot4Battery [commented out] : [favSlot4Sel ==eStateName.FAVORITES_BATTERY ]
                  (junction 9004) -> (junction 9005) [commented out]
                  Flow.ButtonTriggeredMenu.Menu.MainSubMenu.SettingsSubMenu.FavoritesSubMenu.Slot4Menu.Slot4FavoritesMenu1.Slot4Battery -> Flow.ButtonTriggeredMenu.Menu.MainSubMenu.SettingsSubMenu.FavoritesSubMenu.Slot4Menu.Slot4FavoritesMenu1.Slot4WaterTemperature [commented out] : DOWN
                  Flow.ButtonTriggeredMenu.Menu.MainSubMenu.SettingsSubMenu.FavoritesSubMenu.Slot4Menu.Slot4FavoritesMenu1.Slot4WaterTemperature -> Flow.ButtonTriggeredMenu.Menu.MainSubMenu.SettingsSubMenu.FavoritesSubMenu.Slot4Menu.Slot4FavoritesMenu1.Slot4Battery [commented out] : UP
                  (junction 9005) -> Flow.ButtonTriggeredMenu.Menu.MainSubMenu.SettingsSubMenu.FavoritesSubMenu.Slot4Menu.Slot4FavoritesMenu1.Slot4WaterTemperature [commented out] : [favSlot4Sel ==eStateName.FAVORITES_WATER_TEMPERATURE ]
                  (junction 9005) -> (junction 9006) [commented out]
                  Flow.ButtonTriggeredMenu.Menu.MainSubMenu.SettingsSubMenu.FavoritesSubMenu.Slot4Menu.Slot4FavoritesMenu1.Slot4WaterTemperature -> Flow.ButtonTriggeredMenu.Menu.MainSubMenu.SettingsSubMenu.FavoritesSubMenu.Slot4Menu.Slot4FavoritesMenu1.Slot4Odometer [commented out] : DOWN
                  Flow.ButtonTriggeredMenu.Menu.MainSubMenu.SettingsSubMenu.FavoritesSubMenu.Slot4Menu.Slot4FavoritesMenu1.Slot4Odometer -> Flow.ButtonTriggeredMenu.Menu.MainSubMenu.SettingsSubMenu.FavoritesSubMenu.Slot4Menu.Slot4FavoritesMenu1.Slot4WaterTemperature [commented out] : UP
                  (junction 9006) -> Flow.ButtonTriggeredMenu.Menu.MainSubMenu.SettingsSubMenu.FavoritesSubMenu.Slot4Menu.Slot4FavoritesMenu1.Slot4Odometer [commented out] : [favSlot4Sel ==eStateName.FAVORITES_ODOMETER ]
                  (junction 9006) -> (junction 9007) [commented out]
                  Flow.ButtonTriggeredMenu.Menu.MainSubMenu.SettingsSubMenu.FavoritesSubMenu.Slot4Menu.Slot4FavoritesMenu1.Slot4Odometer -> Flow.ButtonTriggeredMenu.Menu.MainSubMenu.SettingsSubMenu.FavoritesSubMenu.Slot4Menu.Slot4FavoritesMenu1.Slot1Connectivity [commented out] : DOWN
                  Flow.ButtonTriggeredMenu.Menu.MainSubMenu.SettingsSubMenu.FavoritesSubMenu.Slot4Menu.Slot4FavoritesMenu1.Slot1Connectivity -> Flow.ButtonTriggeredMenu.Menu.MainSubMenu.SettingsSubMenu.FavoritesSubMenu.Slot4Menu.Slot4FavoritesMenu1.Slot4Odometer [commented out] : UP
                  (junction 9007) -> Flow.ButtonTriggeredMenu.Menu.MainSubMenu.SettingsSubMenu.FavoritesSubMenu.Slot4Menu.Slot4FavoritesMenu1.Slot1Connectivity [commented out]
                  Flow.ButtonTriggeredMenu.Menu.MainSubMenu.SettingsSubMenu.FavoritesSubMenu.Slot4Menu.Slot4FavoritesMenu1.Slot1Connectivity -> (junction 9008) [commented out] : DOWN
                  (junction 9009) -> Flow.ButtonTriggeredMenu.Menu.MainSubMenu.SettingsSubMenu.FavoritesSubMenu.Slot4Menu.Slot4FavoritesMenu1.Slot1Connectivity [commented out]
                6694 -> Slot4FavoritesMenu
                Slot4FavoritesMenu -> 6695 [order 2] : EXIT
                (junction 6693) -> 6695 : {favSlot4Updater(currentState);}
                Slot4FavoritesMenu -> (junction 6693) [order 1] : SET
              FavoritesSlot1 -> 6380 [order 2] : SET
              6381 -> FavoritesSlot1
              6382 -> FavoritesSlotsMenu
              FavoritesSlot2 -> 6383 [order 1] : SET
              6384 -> FavoritesSlot2
              FavoritesSlotsMenu -> 6385 : EXIT
              FavoritesSlot3 -> 6386 [order 3] : SET
              6387 -> FavoritesSlot3
              FavoritesSlot4 -> 6388 [order 2] : SET
              6389 -> FavoritesSlot4
            state QuickSelectorSubMenu
              (history)
              state QuickSelectorSubMenu1
                state QuickSelectorUP
                  en:
                  currentState = eStateName.QUICK_SELECTOR_UP;
                state QuickSelectorDOWN
                  en:
                  currentState = eStateName.QUICK_SELECTOR_DOWN;
                (default) -> QuickSelectorUP
                QuickSelectorUP -> QuickSelectorDOWN [order 2] : DOWN || UP
                QuickSelectorDOWN -> QuickSelectorUP [order 2] : UP || DOWN
              state QuickSelectorUPSubMenu
                (history)
                state QS_UP_SubMenu
                  (history)
                  state QS_UP_RideMode
                    en:
                    currentState = eStateName.QS_UP_RIDE_MODE;
                  state QS_UP_ABSMode
                    en:
                    currentState = eStateName.QS_UP_ABS_MODE;
                  state QS_UP_MTC
                    en:
                    currentState = eStateName.QS_UP_MTC;
                  state QS_UP_TRIP1
                    en:
                    currentState = eStateName.QS_UP_TRIP1;
                  state QS_UP_TRIP2
                    en:
                    currentState = eStateName.QS_UP_TRIP2;
                  state QS_UP_AUDIO
                    en:
                    currentState = eStateName.QS_UP_AUDIO;
                  state QS_UP_NAVIGATION
                    en:
                    currentState = eStateName.QS_UP_NAVIGATION;
                  state QS_UP_HEATEDGRIPS
                    en:
                    currentState = eStateName.QS_UP_HEATEDGRIPS;
                  state QS_UP_CRUISECONTROL
                    en:
                    currentState = eStateName.QS_UP_CRUISECONTROL;
                  state QS_UP_BIKEINFO
                    en:
                    currentState = eStateName.QS_UP_BIKEINFO;
                  (junction 6811) -> (junction 6810)
                  (junction 6810) -> (junction 6815)
                  (junction 6814) -> (junction 6811) [order 2]
                  (default) -> (junction 6812)
                  (junction 6812) -> (junction 6813)
                  (junction 6813) -> QS_UP_RideMode [order 1] : [variant !=eBikeVariant.duke200]
                  (junction 6836) -> (junction 6812)
                  (junction 6813) -> (junction 6818) [order 2]
                  (junction 6814) -> QS_UP_RideMode [order 1] : [variant !=eBikeVariant.duke200]
                  (junction 6817) -> (junction 6814)
                  QS_UP_RideMode -> (junction 6815) [order 2] : UP
                  (junction 6815) -> (junction 6833)
                  QS_UP_RideMode -> QS_UP_ABSMode [order 1] : DOWN
                  QS_UP_ABSMode -> (junction 6817) [order 1] : UP
                  (junction 6818) -> QS_UP_ABSMode
                  (junction 6819) -> QS_UP_ABSMode
                  QS_UP_ABSMode -> (junction 6820) [order 2] : DOWN
                  (junction 6822) -> (junction 6819) [order 2]
                  (junction 6820) -> (junction 6821)
                  QS_UP_MTC -> QS_UP_ABSMode [order 1] : UP
                  (junction 6821) -> QS_UP_MTC [order 1] : [variant !=eBikeVariant.duke200]
                  (junction 6822) -> QS_UP_MTC [order 1] : [variant !=eBikeVariant.duke200]
                  (junction 6821) -> QS_UP_TRIP1 [order 2]
                  (junction 6823) -> (junction 6822)
                  QS_UP_MTC -> QS_UP_TRIP1 [order 2] : DOWN
                  QS_UP_TRIP1 -> (junction 6823) [order 1] : UP
                  QS_UP_TRIP1 -> QS_UP_TRIP2 [order 2] : DOWN
                  QS_UP_TRIP2 -> QS_UP_TRIP1 [order 2] : UP
                  (junction 6824) -> QS_UP_TRIP2
                  QS_UP_AUDIO -> (junction 6824) [order 2] : UP
                  (junction 6826) -> (junction 6824) [order 2]
                  QS_UP_TRIP2 -> (junction 6825) [order 1] : DOWN
                  (junction 6825) -> QS_UP_AUDIO [order 1] : [CCU_Status == eCCUStatus.installed || ...
                      | CCU_Music_Feature != 0 || ...
                      | CCU_BT_Status != 0]
                  (junction 6825) -> (junction 6827) [order 2]
                  (junction 6826) -> QS_UP_AUDIO [order 1] : [CCU_Status == eCCUStatus.installed || ...
                      | CCU_Music_Feature != 0 || ...
                      | CCU_BT_Status != 0]
                  QS_UP_NAVIGATION -> (junction 6826) [order 2] : UP
                  (junction 6828) -> (junction 6826) [order 2]
                  QS_UP_AUDIO -> (junction 6827) [order 1] : DOWN
                  (junction 6827) -> QS_UP_NAVIGATION [order 1] : [CCU_Status == eCCUStatus.installed || ...
                      | CCU_TbT_Navigation_Feature != 0 || ...
                      | CCU_BT_Status != 0]
                  (junction 6827) -> (junction 6829) [order 2]
                  (junction 6828) -> QS_UP_NAVIGATION [order 1] : [CCU_Status == eCCUStatus.installed || ...
                      | CCU_TbT_Navigation_Feature != 0 || ...
                      | CCU_BT_Status != 0]
                  QS_UP_HEATEDGRIPS -> (junction 6828) [order 2] : UP
                  (junction 6830) -> (junction 6828) [order 2]
                  QS_UP_NAVIGATION -> (junction 6829) [order 1] : DOWN
                  (junction 6829) -> QS_UP_HEATEDGRIPS [order 1] : [(Heated_Grips || ...
                      | heatedGrips == eFeatureStatus.available) &&...
                      | variant !=eBikeVariant.duke200]
                  (junction 6829) -> (junction 6831) [order 2]
                  (junction 6830) -> QS_UP_HEATEDGRIPS [order 1] : [(Heated_Grips || ...
                      | heatedGrips == eFeatureStatus.available) &&...
                      | variant !=eBikeVariant.duke200]
                  QS_UP_CRUISECONTROL -> (junction 6830) [order 2] : UP
                  (junction 6832) -> (junction 6830) [order 2]
                  QS_UP_HEATEDGRIPS -> (junction 6831) [order 1] : DOWN
                  (junction 6831) -> QS_UP_CRUISECONTROL [order 1] : [ECU_SL_Feature !=0 && ...
                      | variant !=eBikeVariant.duke200]
                  (junction 6832) -> QS_UP_CRUISECONTROL [order 1] : [ECU_SL_Feature !=0 && ...
                      | variant !=eBikeVariant.duke200]
                  (junction 6831) -> (junction 6834) [order 2]
                  (junction 6835) -> (junction 6832)
                  QS_UP_CRUISECONTROL -> (junction 6834) [order 1] : DOWN
                  (junction 6833) -> (junction 6834)
                  (junction 6834) -> QS_UP_BIKEINFO
                  QS_UP_BIKEINFO -> (junction 6835) [order 1] : UP
                  QS_UP_BIKEINFO -> (junction 6836) [order 2] : DOWN
                (junction 6802) -> 6804 : {quickSelectorUp = currentState;
                    | BLU_FnSetQuickSelectorUP(quickSelectorUp);}
                QS_UP_SubMenu -> 6804 [order 2] : EXIT
                QS_UP_SubMenu -> (junction 6802) [order 1] : SET
                6803 -> QS_UP_SubMenu
              state QuickSelectorDOWNSubMenu
                (history)
                state QS_DOWN_SubMenu
                  (history)
                  state QS_DOWN_RideMode
                    en:
                    currentState = eStateName.QS_DOWN_RIDE_MODE;
                  state QS_DOWN_ABSMode
                    en:
                    currentState = eStateName.QS_DOWN_ABS_MODE;
                  state QS_DOWN_MTC
                    en:
                    currentState = eStateName.QS_DOWN_MTC;
                  state QS_DOWN_TRIP2
                    en:
                    currentState = eStateName.QS_DOWN_TRIP1;
                  state QS_DOWN_TRIP3
                    en:
                    currentState = eStateName.QS_DOWN_TRIP2;
                  state QS_DOWN_AUDIO
                    en:
                    currentState = eStateName.QS_DOWN_AUDIO;
                  state QS_DOWN_NAVIGATION
                    en:
                    currentState = eStateName.QS_DOWN_NAVIGATION;
                  state QS_DOWN_HEATEDGRIPS
                    en:
                    currentState = eStateName.QS_DOWN_HEATEDGRIPS;
                  state QS_DOWN_CRUISECONTROL
                    en:
                    currentState = eStateName.QS_DOWN_CRUISECONTROL;
                  state QS_DOWN_BIKEINFO
                    en:
                    currentState = eStateName.QS_DOWN_BIKEINFO;
                  (junction 6917) -> (junction 6916)
                  (junction 6916) -> (junction 6921)
                  (junction 6920) -> (junction 6917) [order 2]
                  (junction 6918) -> (junction 6919)
                  (junction 6919) -> QS_DOWN_RideMode [order 1] : [variant !=eBikeVariant.duke200]
                  (default) -> (junction 6918)
                  (junction 6941) -> (junction 6918)
                  (junction 6919) -> (junction 6923) [order 2]
                  (junction 6920) -> QS_DOWN_RideMode [order 1] : [variant !=eBikeVariant.duke200]
                  QS_DOWN_RideMode -> (junction 6921) [order 2] : UP
                  (junction 6922) -> (junction 6920)
                  (junction 6921) -> (junction 6938)
                  QS_DOWN_RideMode -> QS_DOWN_ABSMode [order 1] : DOWN
                  QS_DOWN_ABSMode -> (junction 6922) [order 1] : UP
                  (junction 6923) -> QS_DOWN_ABSMode
                  (junction 6924) -> QS_DOWN_ABSMode
                  QS_DOWN_ABSMode -> (junction 6925) [order 2] : DOWN
                  (junction 6927) -> (junction 6924) [order 2]
                  (junction 6925) -> (junction 6926)
                  QS_DOWN_MTC -> QS_DOWN_ABSMode [order 1] : UP
                  (junction 6926) -> QS_DOWN_MTC [order 1] : [variant !=eBikeVariant.duke200]
                  (junction 6926) -> QS_DOWN_TRIP2 [order 2]
                  (junction 6927) -> QS_DOWN_MTC [order 1] : [variant !=eBikeVariant.duke200]
                  (junction 6928) -> (junction 6927)
                  QS_DOWN_MTC -> QS_DOWN_TRIP2 [order 2] : DOWN
                  QS_DOWN_TRIP2 -> (junction 6928) [order 1] : UP
                  QS_DOWN_TRIP2 -> QS_DOWN_TRIP3 [order 2] : DOWN
                  QS_DOWN_TRIP3 -> QS_DOWN_TRIP2 [order 2] : UP
                  (junction 6929) -> QS_DOWN_TRIP3
                  QS_DOWN_AUDIO -> (junction 6929) [order 2] : UP
                  (junction 6931) -> (junction 6929) [order 2]
                  QS_DOWN_TRIP3 -> (junction 6930) [order 1] : DOWN
                  (junction 6930) -> QS_DOWN_AUDIO [order 1] : [CCU_Status == eCCUStatus.installed || ...
                      | CCU_Music_Feature != 0 || ...
                      | CCU_BT_Status != 0]
                  (junction 6930) -> (junction 6932) [order 2]
                  (junction 6931) -> QS_DOWN_AUDIO [order 1] : [CCU_Status == eCCUStatus.installed || ...
                      | CCU_Music_Feature != 0 || ...
                      | CCU_BT_Status != 0]
                  QS_DOWN_NAVIGATION -> (junction 6931) [order 2] : UP
                  (junction 6933) -> (junction 6931) [order 2]
                  QS_DOWN_AUDIO -> (junction 6932) [order 1] : DOWN
                  (junction 6932) -> QS_DOWN_NAVIGATION [order 1] : [CCU_Status == eCCUStatus.installed || ...
                      | CCU_TbT_Navigation_Feature != 0 || ...
                      | CCU_BT_Status != 0]
                  (junction 6932) -> (junction 6934) [order 2]
                  (junction 6933) -> QS_DOWN_NAVIGATION [order 1] : [CCU_Status == eCCUStatus.installed || ...
                      | CCU_TbT_Navigation_Feature != 0 || ...
                      | CCU_BT_Status != 0]
                  QS_DOWN_HEATEDGRIPS -> (junction 6933) [order 2] : UP
                  (junction 6935) -> (junction 6933) [order 2]
                  QS_DOWN_NAVIGATION -> (junction 6934) [order 1] : DOWN
                  (junction 6934) -> QS_DOWN_HEATEDGRIPS [order 1] : [(Heated_Grips || ...
                      | heatedGrips == eFeatureStatus.available) &&...
                      | variant !=eBikeVariant.duke200]
                  (junction 6934) -> (junction 6936) [order 2]
                  (junction 6935) -> QS_DOWN_HEATEDGRIPS [order 1] : [variant !=eBikeVariant.duke200 && ...
                      | (Heated_Grips || ...
                      | heatedGrips == eFeatureStatus.available)]
                  QS_DOWN_CRUISECONTROL -> (junction 6935) [order 2] : UP
                  (junction 6937) -> (junction 6935) [order 2]
                  QS_DOWN_HEATEDGRIPS -> (junction 6936) [order 1] : DOWN
                  (junction 6936) -> QS_DOWN_CRUISECONTROL [order 1] : [ECU_SL_Feature !=0 && ...
                      | variant !=eBikeVariant.duke200]
                  (junction 6937) -> QS_DOWN_CRUISECONTROL [order 1] : [ECU_SL_Feature !=0 && ...
                      | variant !=eBikeVariant.duke200]
                  (junction 6936) -> (junction 6939) [order 2]
                  (junction 6940) -> (junction 6937)
                  QS_DOWN_CRUISECONTROL -> (junction 6939) [order 1] : DOWN
                  (junction 6938) -> (junction 6939)
                  (junction 6939) -> QS_DOWN_BIKEINFO
                  QS_DOWN_BIKEINFO -> (junction 6940) [order 1] : UP
                  QS_DOWN_BIKEINFO -> (junction 6941) [order 2] : DOWN